simulink model "neuronchainv9"  (Simulink 7.6)
settings: Solver=ode45  StartTime=0.0  StopTime=250  RelTol=1e-3
values from neighbouring files (symbols this model uses):
  alpha = 0.8    [excneuroninit.m]
  amp = 1    [excneuroninit.m]
  ampe = 0.5    [excneuroninit.m]
  beta = 0.05    [excneuroninit.m]
  h = -0.5    [excneuroninit.m]
  inittime = 0.1    [excneuroninit.m]
  maxcon = 10    [excneuroninit.m]
  neurodist = 1    [excneuroninit.m]
  offsetf = 4    [excneuroninit.m]
  offseth = 4    [excneuroninit.m]
  offsetvali = 0    [excneuroninit.m]
  sensoryamp = 0.5    [excneuroninit.m]
  sigslope = 3    [excneuroninit.m]
  sigslopef = 1    [excneuroninit.m]
  sigslopei = 3    [excneuroninit.m]
  tau_s = 5    [excneuroninit.m]
  tau_u = 0.05    [excneuroninit.m]
  tau_v = 0.1    [excneuroninit.m]
  vari = 0.5    [excneuroninit.m]
undefined symbols (not defined in the file or in its folder): sigslopecal
libraries: simulink x560
(part 2 of 21)

== system neuronchainv9/signalsplit1k ==
  Mux : Mux  Inputs=5
  Pulse Generator{1..5} (5 alike) : DiscretePulseGenerator  PulseType=Time based  Period=4  PulseWidth=25  PhaseDelay=0.75
  Random Number{1..5} (5 alike) : RandomNumber  Variance=vari  SampleTime=0.1
  Sum{1..5} (5 alike) : Sum  Inputs=|++
  Out1 : Outport
  wires:
  Random Number1/1 -> Sum1/1
  Pulse Generator1/1 -> Sum1/2
  Random Number2/1 -> Sum2/1
  Pulse Generator2/1 -> Sum2/2
  Random Number3/1 -> Sum3/1
  Pulse Generator3/1 -> Sum3/2
  Random Number4/1 -> Sum4/1
  Pulse Generator4/1 -> Sum4/2
  Random Number5/1 -> Sum5/1
  Pulse Generator5/1 -> Sum5/2
  Sum5/1 -> Mux/1
  Sum2/1 -> Mux/2
  Sum1/1 -> Mux/3
  Sum4/1 -> Mux/4
  Sum3/1 -> Mux/5
  Mux/1 -> Out1/1

== system neuronchainv9/signalsplit2 ==
  Mux : Mux  Inputs=5
  Pulse Generator{1..5} (5 alike) : DiscretePulseGenerator  PulseType=Time based  Period=4  PulseWidth=25  PhaseDelay=1.35
  Random Number{1..5} (5 alike) : RandomNumber  Variance=vari  SampleTime=0.1
  Sum{1..5} (5 alike) : Sum  Inputs=|++
  Out1 : Outport
  wires:
  Pulse Generator2/1 -> Sum2/2
  Random Number2/1 -> Sum2/1
  Pulse Generator1/1 -> Sum1/2
  Random Number1/1 -> Sum1/1
  Pulse Generator3/1 -> Sum3/2
  Random Number3/1 -> Sum3/1
  Pulse Generator4/1 -> Sum4/2
  Random Number4/1 -> Sum4/1
  Pulse Generator5/1 -> Sum5/2
  Random Number5/1 -> Sum5/1
  Sum2/1 -> Mux/1
  Sum3/1 -> Mux/2
  Sum5/1 -> Mux/3
  Sum1/1 -> Mux/4
  Sum4/1 -> Mux/5
  Mux/1 -> Out1/1

== system neuronchainv9/signalsplit2k ==
  Mux : Mux  Inputs=5
  Pulse Generator{1..5} (5 alike) : DiscretePulseGenerator  PulseType=Time based  Period=4  PulseWidth=25  PhaseDelay=2.75
  Random Number{1..5} (5 alike) : RandomNumber  Variance=vari  SampleTime=0.1
  Sum{1..5} (5 alike) : Sum  Inputs=|++
  Out1 : Outport
  wires:
  Pulse Generator2/1 -> Sum2/2
  Random Number2/1 -> Sum2/1
  Pulse Generator1/1 -> Sum1/2
  Random Number1/1 -> Sum1/1
  Pulse Generator3/1 -> Sum3/2
  Random Number3/1 -> Sum3/1
  Pulse Generator4/1 -> Sum4/2
  Random Number4/1 -> Sum4/1
  Pulse Generator5/1 -> Sum5/2
  Random Number5/1 -> Sum5/1
  Sum2/1 -> Mux/1
  Sum3/1 -> Mux/2
  Sum5/1 -> Mux/3
  Sum1/1 -> Mux/4
  Sum4/1 -> Mux/5
  Mux/1 -> Out1/1

== system neuronchainv9/EAT_POOL/Subsystem ==
  port1 : Inport
  port2 : Inport  Port=2
  Add : Sum  Inputs=-+
  Demux : Demux  Outputs=2
  Gain : Gain  Gain=1/tau_v
  Integrator : Integrator  InitialCondition=1
  MATLAB Fcn : MATLABFcn  MATLABFcn=ampe*exp(-1*(u-neurodist).*(u-neurodist)/18)  OutputDimensions=maxcon
  Product1 : Product  RndMeth=Zero
  Product2 : Product  RndMeth=Zero
  Sigmoid1 : Subsystem  TreatAsAtomicUnit=on  mask{slope=sigslope, offset=offsetvali}
      mask help: Created by Han, Cheol
      mask Display: plot([10 15 20 25 30 35 40 45 50], [0 0.01 0.1 0.2 0.5 0.8 0.9 0.99 1])
  Sigmoid2 : Subsystem  TreatAsAtomicUnit=on  mask{slope=sigslope, offset=offsetvali}
      mask help: Created by Han, Cheol
      mask Display: plot([10 15 20 25 30 35 40 45 50], [0 0.01 0.1 0.2 0.5 0.8 0.9 0.99 1])
  Sum2 : Sum  Inputs=+  SaturateOnIntegerOverflow=on
  Out2 : Outport
  wires:
  Product2/1 -> Add/2
  port2/1 -> Sigmoid2/1(x)
  port1/1 -> MATLAB Fcn/1
  Sigmoid1/1(y) -> Product2/2
  Sum2/1 -> Product2/1
  Product1/1 -> Sum2/1
  Sigmoid2/1(y) -> Product1/2
  MATLAB Fcn/1 -> Product1/1
  Integrator/1 -> Out2/1, Sigmoid1/1(x), Add/1
  Gain/1 -> Integrator/1
  Add/1 -> Gain/1

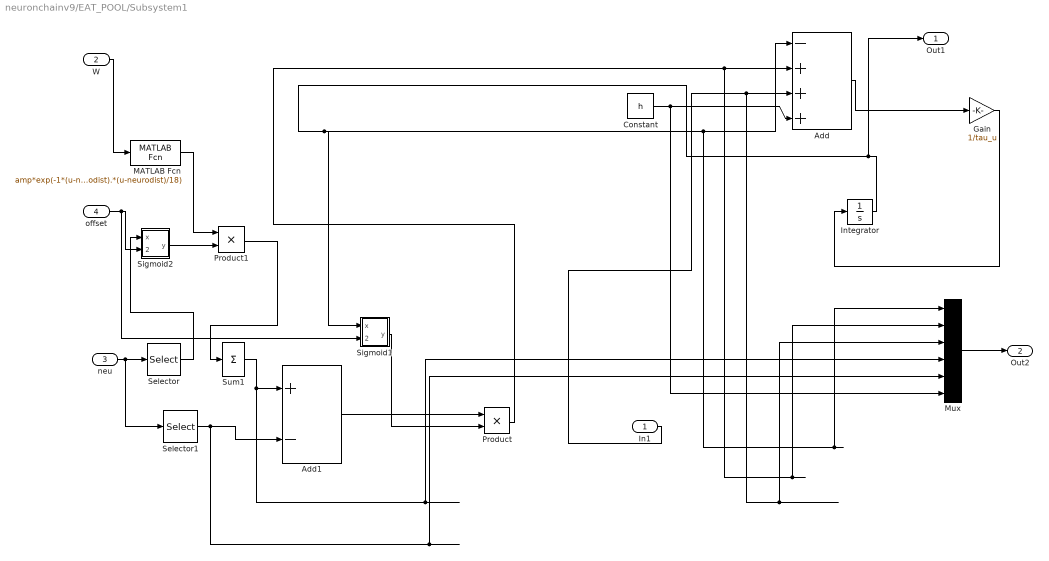
== system neuronchainv9/EAT_POOL/Subsystem1 ==
  In1 : Inport
  W : Inport  Port=2
  neu : Inport  Port=3
  offset : Inport  Port=4
  Add : Sum  Inputs=-+++
  Add1 : Sum  Inputs=+-
  Constant : Constant  Value=h
  Gain : Gain  Gain=1/tau_u
  Integrator : Integrator  InitialCondition=1
  MATLAB Fcn : MATLABFcn  MATLABFcn=amp*exp(-1*(u-neurodist).*(u-neurodist)/18)  OutputDimensions=maxcon
  Mux : Mux  Inputs=6
  Product : Product  RndMeth=Zero
  Product1 : Product  RndMeth=Zero
  Selector : Selector  InputPortWidth=maxcon+1  IndexOptions=Starting index (dialog)  Indices=2  OutputSizes=maxcon
  Selector1 : Selector  InputPortWidth=maxcon+1  IndexOptions=Starting index (dialog)  Indices=1  OutputSizes=1
  Sigmoid1 : Subsystem  TreatAsAtomicUnit=on  mask{slope=sigslope}
      mask help: Created by Han, Cheol
      mask Display: plot([10 15 20 25 30 35 40 45 50], [0 0.01 0.1 0.2 0.5 0.8 0.9 0.99 1])
  Sigmoid2 : Subsystem  TreatAsAtomicUnit=on  mask{slope=sigslope}
      mask help: Created by Han, Cheol
      mask Display: plot([10 15 20 25 30 35 40 45 50], [0 0.01 0.1 0.2 0.5 0.8 0.9 0.99 1])
  Sum1 : Sum  Inputs=+  SaturateOnIntegerOverflow=on
  Out1 : Outport
  Out2 : Outport  Port=2
  wires:
  Mux/1 -> Out2/1
  Constant/1 -> Mux/6, Add/4
  Add1/1 -> Product/2
  Sigmoid2/1(y) -> Product1/2
  Selector1/1 -> Mux/5, Add1/2
  Sum1/1 -> Mux/4, Add1/1
  MATLAB Fcn/1 -> Product1/1
  W/1 -> MATLAB Fcn/1
  Selector/1 -> Sigmoid2/1(x)
  Product1/1 -> Sum1/1
  neu/1 -> Selector1/1, Selector/1
  In1/1 -> Mux/3, Add/3
  Product/1 -> Mux/2, Add/2
  Sigmoid1/1(y) -> Product/1
  Integrator/1 -> Out1/1, Add/1, Mux/1, Sigmoid1/1(x)
  Gain/1 -> Integrator/1
  Add/1 -> Gain/1
  offset/1 -> Sigmoid2/2(offset), Sigmoid1/2(offset)

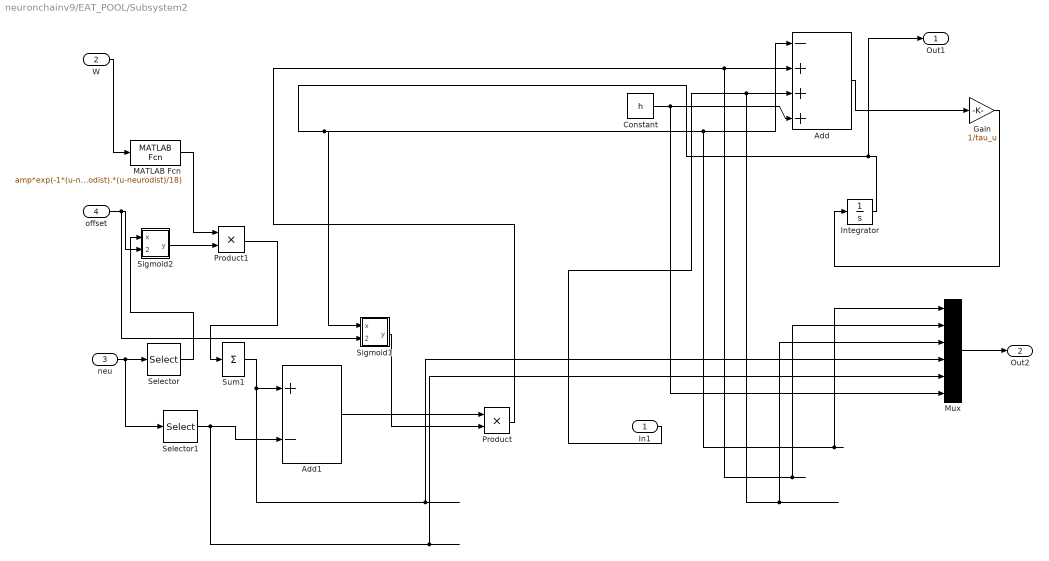
== system neuronchainv9/EAT_POOL/Subsystem2 ==
  In1 : Inport
  W : Inport  Port=2
  neu : Inport  Port=3
  offset : Inport  Port=4
  Add : Sum  Inputs=-+++
  Add1 : Sum  Inputs=+-
  Constant : Constant  Value=h
  Gain : Gain  Gain=1/tau_u
  Integrator : Integrator  InitialCondition=1
  MATLAB Fcn : MATLABFcn  MATLABFcn=amp*exp(-1*(u-neurodist).*(u-neurodist)/18)  OutputDimensions=maxcon
  Mux : Mux  Inputs=6
  Product : Product  RndMeth=Zero
  Product1 : Product  RndMeth=Zero
  Selector : Selector  InputPortWidth=maxcon+1  IndexOptions=Starting index (dialog)  Indices=2  OutputSizes=maxcon
  Selector1 : Selector  InputPortWidth=maxcon+1  IndexOptions=Starting index (dialog)  Indices=1  OutputSizes=1
  Sigmoid1 : Subsystem  TreatAsAtomicUnit=on  mask{slope=sigslope}
      mask help: Created by Han, Cheol
      mask Display: plot([10 15 20 25 30 35 40 45 50], [0 0.01 0.1 0.2 0.5 0.8 0.9 0.99 1])
  Sigmoid2 : Subsystem  TreatAsAtomicUnit=on  mask{slope=sigslope}
      mask help: Created by Han, Cheol
      mask Display: plot([10 15 20 25 30 35 40 45 50], [0 0.01 0.1 0.2 0.5 0.8 0.9 0.99 1])
  Sum1 : Sum  Inputs=+  SaturateOnIntegerOverflow=on
  Out1 : Outport
  Out2 : Outport  Port=2
  wires:
  Mux/1 -> Out2/1
  Constant/1 -> Mux/6, Add/4
  Add1/1 -> Product/2
  Sigmoid2/1(y) -> Product1/2
  Selector1/1 -> Mux/5, Add1/2
  Sum1/1 -> Mux/4, Add1/1
  MATLAB Fcn/1 -> Product1/1
  W/1 -> MATLAB Fcn/1
  Selector/1 -> Sigmoid2/1(x)
  Product1/1 -> Sum1/1
  neu/1 -> Selector1/1, Selector/1
  In1/1 -> Mux/3, Add/3
  Product/1 -> Mux/2, Add/2
  Sigmoid1/1(y) -> Product/1
  Integrator/1 -> Out1/1, Add/1, Mux/1, Sigmoid1/1(x)
  Gain/1 -> Integrator/1
  Add/1 -> Gain/1
  offset/1 -> Sigmoid2/2(offset), Sigmoid1/2(offset)

== system neuronchainv9/EAT_POOL/Subsystem3 ==
  In1 : Inport
  W : Inport  Port=2
  neu : Inport  Port=3
  offset : Inport  Port=4
  Add : Sum  Inputs=-+++
  Add1 : Sum  Inputs=+-
  Constant : Constant  Value=h
  Gain : Gain  Gain=1/tau_u
  Integrator : Integrator  InitialCondition=1
  MATLAB Fcn : MATLABFcn  MATLABFcn=amp*exp(-1*(u-neurodist).*(u-neurodist)/18)  OutputDimensions=maxcon
  Mux : Mux  Inputs=6
  Product : Product  RndMeth=Zero
  Product1 : Product  RndMeth=Zero
  Selector : Selector  InputPortWidth=maxcon+1  IndexOptions=Starting index (dialog)  Indices=2  OutputSizes=maxcon
  Selector1 : Selector  InputPortWidth=maxcon+1  IndexOptions=Starting index (dialog)  Indices=1  OutputSizes=1
  Sigmoid1 : Subsystem  TreatAsAtomicUnit=on  mask{slope=sigslope}
      mask help: Created by Han, Cheol
      mask Display: plot([10 15 20 25 30 35 40 45 50], [0 0.01 0.1 0.2 0.5 0.8 0.9 0.99 1])
  Sigmoid2 : Subsystem  TreatAsAtomicUnit=on  mask{slope=sigslope}
      mask help: Created by Han, Cheol
      mask Display: plot([10 15 20 25 30 35 40 45 50], [0 0.01 0.1 0.2 0.5 0.8 0.9 0.99 1])
  Sum1 : Sum  Inputs=+  SaturateOnIntegerOverflow=on
  Out1 : Outport
  Out2 : Outport  Port=2
  wires:
  Mux/1 -> Out2/1
  Constant/1 -> Mux/6, Add/4
  Add1/1 -> Product/2
  Sigmoid2/1(y) -> Product1/2
  Selector1/1 -> Mux/5, Add1/2
  Sum1/1 -> Mux/4, Add1/1
  MATLAB Fcn/1 -> Product1/1
  W/1 -> MATLAB Fcn/1
  Selector/1 -> Sigmoid2/1(x)
  Product1/1 -> Sum1/1
  neu/1 -> Selector1/1, Selector/1
  In1/1 -> Mux/3, Add/3
  Product/1 -> Mux/2, Add/2
  Sigmoid1/1(y) -> Product/1
  Integrator/1 -> Out1/1, Add/1, Mux/1, Sigmoid1/1(x)
  Gain/1 -> Integrator/1
  Add/1 -> Gain/1
  offset/1 -> Sigmoid2/2(offset), Sigmoid1/2(offset)

== system neuronchainv9/EAT_POOL/Subsystem4 ==
  port1 : Inport
  port2 : Inport  Port=2
  Add : Sum  Inputs=-+
  Gain : Gain  Gain=1/tau_v
  Integrator : Integrator  InitialCondition=1
  MATLAB Fcn : MATLABFcn  MATLABFcn=ampe*exp(-1*(u-neurodist).*(u-neurodist)/18)  OutputDimensions=maxcon
  Product1 : Product  RndMeth=Zero
  Product2 : Product  RndMeth=Zero
  Sigmoid1 : Subsystem  TreatAsAtomicUnit=on  mask{slope=sigslope, offset=offsetvali}
      mask help: Created by Han, Cheol
      mask Display: plot([10 15 20 25 30 35 40 45 50], [0 0.01 0.1 0.2 0.5 0.8 0.9 0.99 1])
  Sigmoid2 : Subsystem  TreatAsAtomicUnit=on  mask{slope=sigslope, offset=offsetvali}
      mask help: Created by Han, Cheol
      mask Display: plot([10 15 20 25 30 35 40 45 50], [0 0.01 0.1 0.2 0.5 0.8 0.9 0.99 1])
  Sum2 : Sum  Inputs=+  SaturateOnIntegerOverflow=on
  Out2 : Outport
  wires:
  Product2/1 -> Add/2
  port2/1 -> Sigmoid2/1(x)
  port1/1 -> MATLAB Fcn/1
  Sigmoid1/1(y) -> Product2/2
  Sum2/1 -> Product2/1
  Product1/1 -> Sum2/1
  Sigmoid2/1(y) -> Product1/2
  MATLAB Fcn/1 -> Product1/1
  Integrator/1 -> Out2/1, Sigmoid1/1(x), Add/1
  Gain/1 -> Integrator/1
  Add/1 -> Gain/1

== system neuronchainv9/EAT_POOL/Subsystem5 ==
  port1 : Inport
  port2 : Inport  Port=2
  Add : Sum  Inputs=-+
  Gain : Gain  Gain=1/tau_v
  Integrator : Integrator  InitialCondition=1
  MATLAB Fcn : MATLABFcn  MATLABFcn=ampe*exp(-1*(u-neurodist).*(u-neurodist)/18)  OutputDimensions=maxcon
  Product1 : Product  RndMeth=Zero
  Product2 : Product  RndMeth=Zero
  Sigmoid1 : Subsystem  TreatAsAtomicUnit=on  mask{slope=sigslope, offset=offsetvali}
      mask help: Created by Han, Cheol
      mask Display: plot([10 15 20 25 30 35 40 45 50], [0 0.01 0.1 0.2 0.5 0.8 0.9 0.99 1])
  Sigmoid2 : Subsystem  TreatAsAtomicUnit=on  mask{slope=sigslope, offset=offsetvali}
      mask help: Created by Han, Cheol
      mask Display: plot([10 15 20 25 30 35 40 45 50], [0 0.01 0.1 0.2 0.5 0.8 0.9 0.99 1])
  Sum2 : Sum  Inputs=+  SaturateOnIntegerOverflow=on
  Out2 : Outport
  wires:
  Product2/1 -> Add/2
  port2/1 -> Sigmoid2/1(x)
  port1/1 -> MATLAB Fcn/1
  Sigmoid1/1(y) -> Product2/2
  Sum2/1 -> Product2/1
  Product1/1 -> Sum2/1
  Sigmoid2/1(y) -> Product1/2
  MATLAB Fcn/1 -> Product1/1
  Integrator/1 -> Out2/1, Sigmoid1/1(x), Add/1
  Gain/1 -> Integrator/1
  Add/1 -> Gain/1

== system neuronchainv9/EAT_POOL/Subsystem6 ==
  In1 : Inport
  W : Inport  Port=2
  neu : Inport  Port=3
  offset : Inport  Port=4
  Add : Sum  Inputs=-+++
  Add1 : Sum  Inputs=+-
  Constant : Constant  Value=h
  Gain : Gain  Gain=1/tau_u
  Integrator : Integrator  InitialCondition=1
  MATLAB Fcn : MATLABFcn  MATLABFcn=amp*exp(-1*(u-neurodist).*(u-neurodist)/18)  OutputDimensions=maxcon
  Mux : Mux  Inputs=6
  Product : Product  RndMeth=Zero
  Product1 : Product  RndMeth=Zero
  Selector : Selector  InputPortWidth=maxcon+1  IndexOptions=Starting index (dialog)  Indices=2  OutputSizes=maxcon
  Selector1 : Selector  InputPortWidth=maxcon+1  IndexOptions=Starting index (dialog)  Indices=1  OutputSizes=1
  Sigmoid1 : Subsystem  TreatAsAtomicUnit=on  mask{slope=sigslope}
      mask help: Created by Han, Cheol
      mask Display: plot([10 15 20 25 30 35 40 45 50], [0 0.01 0.1 0.2 0.5 0.8 0.9 0.99 1])
  Sigmoid2 : Subsystem  TreatAsAtomicUnit=on  mask{slope=sigslope}
      mask help: Created by Han, Cheol
      mask Display: plot([10 15 20 25 30 35 40 45 50], [0 0.01 0.1 0.2 0.5 0.8 0.9 0.99 1])
  Sum1 : Sum  Inputs=+  SaturateOnIntegerOverflow=on
  Out1 : Outport
  Out2 : Outport  Port=2
  wires:
  Mux/1 -> Out2/1
  Constant/1 -> Mux/6, Add/4
  Add1/1 -> Product/2
  Sigmoid2/1(y) -> Product1/2
  Selector1/1 -> Mux/5, Add1/2
  Sum1/1 -> Mux/4, Add1/1
  MATLAB Fcn/1 -> Product1/1
  W/1 -> MATLAB Fcn/1
  Selector/1 -> Sigmoid2/1(x)
  Product1/1 -> Sum1/1
  neu/1 -> Selector1/1, Selector/1
  In1/1 -> Mux/3, Add/3
  Product/1 -> Mux/2, Add/2
  Sigmoid1/1(y) -> Product/1
  Integrator/1 -> Out1/1, Add/1, Mux/1, Sigmoid1/1(x)
  Gain/1 -> Integrator/1
  Add/1 -> Gain/1
  offset/1 -> Sigmoid2/2(offset), Sigmoid1/2(offset)

== system neuronchainv9/EAT_POOL/Subsystem7 ==
  In1 : Inport
  W : Inport  Port=2
  neu : Inport  Port=3
  offset : Inport  Port=4
  Add : Sum  Inputs=-+++
  Add1 : Sum  Inputs=+-
  Constant : Constant  Value=h
  Gain : Gain  Gain=1/tau_u
  Integrator : Integrator  InitialCondition=1
  MATLAB Fcn : MATLABFcn  MATLABFcn=amp*exp(-1*(u-neurodist).*(u-neurodist)/18)  OutputDimensions=maxcon
  Mux : Mux  Inputs=6
  Product : Product  RndMeth=Zero
  Product1 : Product  RndMeth=Zero
  Selector : Selector  InputPortWidth=maxcon+1  IndexOptions=Starting index (dialog)  Indices=2  OutputSizes=maxcon
  Selector1 : Selector  InputPortWidth=maxcon+1  IndexOptions=Starting index (dialog)  Indices=1  OutputSizes=1
  Sigmoid1 : Subsystem  TreatAsAtomicUnit=on  mask{slope=sigslope}
      mask help: Created by Han, Cheol
      mask Display: plot([10 15 20 25 30 35 40 45 50], [0 0.01 0.1 0.2 0.5 0.8 0.9 0.99 1])
  Sigmoid2 : Subsystem  TreatAsAtomicUnit=on  mask{slope=sigslope}
      mask help: Created by Han, Cheol
      mask Display: plot([10 15 20 25 30 35 40 45 50], [0 0.01 0.1 0.2 0.5 0.8 0.9 0.99 1])
  Sum1 : Sum  Inputs=+  SaturateOnIntegerOverflow=on
  Out1 : Outport
  Out2 : Outport  Port=2
  wires:
  Mux/1 -> Out2/1
  Constant/1 -> Mux/6, Add/4
  Add1/1 -> Product/2
  Sigmoid2/1(y) -> Product1/2
  Selector1/1 -> Mux/5, Add1/2
  Sum1/1 -> Mux/4, Add1/1
  MATLAB Fcn/1 -> Product1/1
  W/1 -> MATLAB Fcn/1
  Selector/1 -> Sigmoid2/1(x)
  Product1/1 -> Sum1/1
  neu/1 -> Selector1/1, Selector/1
  In1/1 -> Mux/3, Add/3
  Product/1 -> Mux/2, Add/2
  Sigmoid1/1(y) -> Product/1
  Integrator/1 -> Out1/1, Add/1, Mux/1, Sigmoid1/1(x)
  Gain/1 -> Integrator/1
  Add/1 -> Gain/1
  offset/1 -> Sigmoid2/2(offset), Sigmoid1/2(offset)

== system neuronchainv9/EAT_POOL/Subsystem8 ==
  port1 : Inport
  port2 : Inport  Port=2
  Add : Sum  Inputs=-+
  Gain : Gain  Gain=1/tau_v
  Integrator : Integrator  InitialCondition=1
  MATLAB Fcn : MATLABFcn  MATLABFcn=ampe*exp(-1*(u-neurodist).*(u-neurodist)/18)  OutputDimensions=maxcon
  Product1 : Product  RndMeth=Zero
  Product2 : Product  RndMeth=Zero
  Sigmoid1 : Subsystem  TreatAsAtomicUnit=on  mask{slope=sigslope, offset=offsetvali}
      mask help: Created by Han, Cheol
      mask Display: plot([10 15 20 25 30 35 40 45 50], [0 0.01 0.1 0.2 0.5 0.8 0.9 0.99 1])
  Sigmoid2 : Subsystem  TreatAsAtomicUnit=on  mask{slope=sigslope, offset=offsetvali}
      mask help: Created by Han, Cheol
      mask Display: plot([10 15 20 25 30 35 40 45 50], [0 0.01 0.1 0.2 0.5 0.8 0.9 0.99 1])
  Sum2 : Sum  Inputs=+  SaturateOnIntegerOverflow=on
  Out2 : Outport
  wires:
  Product2/1 -> Add/2
  port2/1 -> Sigmoid2/1(x)
  port1/1 -> MATLAB Fcn/1
  Sigmoid1/1(y) -> Product2/2
  Sum2/1 -> Product2/1
  Product1/1 -> Sum2/1
  Sigmoid2/1(y) -> Product1/2
  MATLAB Fcn/1 -> Product1/1
  Integrator/1 -> Out2/1, Sigmoid1/1(x), Add/1
  Gain/1 -> Integrator/1
  Add/1 -> Gain/1

== system neuronchainv9/EAT_POOL/Subsystem9 ==
  port1 : Inport
  port2 : Inport  Port=2
  Add : Sum  Inputs=-+
  Gain : Gain  Gain=1/tau_v
  Integrator : Integrator  InitialCondition=1
  MATLAB Fcn : MATLABFcn  MATLABFcn=ampe*exp(-1*(u-neurodist).*(u-neurodist)/18)  OutputDimensions=maxcon
  Product1 : Product  RndMeth=Zero
  Product2 : Product  RndMeth=Zero
  Sigmoid1 : Subsystem  TreatAsAtomicUnit=on  mask{slope=sigslope, offset=offsetvali}
      mask help: Created by Han, Cheol
      mask Display: plot([10 15 20 25 30 35 40 45 50], [0 0.01 0.1 0.2 0.5 0.8 0.9 0.99 1])
  Sigmoid2 : Subsystem  TreatAsAtomicUnit=on  mask{slope=sigslope, offset=offsetvali}
      mask help: Created by Han, Cheol
      mask Display: plot([10 15 20 25 30 35 40 45 50], [0 0.01 0.1 0.2 0.5 0.8 0.9 0.99 1])
  Sum2 : Sum  Inputs=+  SaturateOnIntegerOverflow=on
  Out2 : Outport
  wires:
  Product2/1 -> Add/2
  port2/1 -> Sigmoid2/1(x)
  port1/1 -> MATLAB Fcn/1
  Sigmoid1/1(y) -> Product2/2
  Sum2/1 -> Product2/1
  Product1/1 -> Sum2/1
  Sigmoid2/1(y) -> Product1/2
  MATLAB Fcn/1 -> Product1/1
  Integrator/1 -> Out2/1, Sigmoid1/1(x), Add/1
  Gain/1 -> Integrator/1
  Add/1 -> Gain/1

== system neuronchainv9/Hebbconp0to1/  ==
  ui : Inport
  uj : Inport  Port=2
  Sij : Inport  Port=3
  1_over_taus : Gain  Gain=1/tau_s
  Clock : Clock
      settings: Decimation=10
  Constant : Constant  Value=alpha
  Constant3 : Constant  Value=inittime
  Integrator : Integrator
  Product : Product  Inputs=3  RndMeth=Zero
  Product1 : Product  RndMeth=Zero
  Sum : Sum  Inputs=|+-
  Sum1 : Sum  Inputs=|+-
  Sum2 : Sum  Inputs=+-|
  Switch1 : Switch  Criteria=u2 > Threshold
  beta : Gain  Gain=beta
  f(ui) : Subsystem  TreatAsAtomicUnit=on  mask{slope=sigslopef, offset=offsetf}
      mask help: Created by Han, Cheol
      mask Display: plot([10 15 20 25 30 35 40 45 50], [0 0.01 0.1 0.2 0.5 0.8 0.9 0.99 1])
  f(uj) : Subsystem  TreatAsAtomicUnit=on  mask{slope=sigslopef, offset=offsetf}
      mask help: Created by Han, Cheol
      mask Display: plot([10 15 20 25 30 35 40 45 50], [0 0.01 0.1 0.2 0.5 0.8 0.9 0.99 1])
  h(ui) : Subsystem  TreatAsAtomicUnit=on  mask{slope=sigslopei, offset=offseth}
      mask help: Created by Han, Cheol
      mask Display: plot([10 15 20 25 30 35 40 45 50], [0 0.01 0.1 0.2 0.5 0.8 0.9 0.99 1])
  Out1 : Outport
  wires:
  ui/1 -> h(ui)/1(x), f(ui)/1(x)
  uj/1 -> f(uj)/1(x)
  f(ui)/1(y) -> Product/1
  f(uj)/1(y) -> Product/2
  Constant/1 -> Product/3
  Product/1 -> Sum/1
  Sum/1 -> Product1/2
  h(ui)/1(y) -> Product1/1
  Product1/1 -> Sum1/1
  beta/1 -> Sum1/2
  Sum1/1 -> 1_over_taus/1
  1_over_taus/1 -> Integrator/1
  Integrator/1 -> Switch1/1, Sum/2
  Sum2/1 -> Switch1/2
  Clock/1 -> Sum2/1
  Constant3/1 -> Sum2/2
  Switch1/1 -> beta/1, Out1/1
  Sij/1 -> Switch1/3

== system neuronchainv9/Hebbconp0to1/ 2 ==
  ui : Inport
  uj : Inport  Port=2
  Sij : Inport  Port=3
  1_over_taus : Gain  Gain=1/tau_s
  Clock : Clock
      settings: Decimation=10
  Constant : Constant  Value=alpha
  Constant3 : Constant  Value=inittime
  Integrator : Integrator
  Product : Product  Inputs=3  RndMeth=Zero
  Product1 : Product  RndMeth=Zero
  Sum : Sum  Inputs=|+-
  Sum1 : Sum  Inputs=|+-
  Sum2 : Sum  Inputs=+-|
  Switch1 : Switch  Criteria=u2 > Threshold
  beta : Gain  Gain=beta
  f(ui) : Subsystem  TreatAsAtomicUnit=on  mask{slope=sigslopef, offset=offsetf}
      mask help: Created by Han, Cheol
      mask Display: plot([10 15 20 25 30 35 40 45 50], [0 0.01 0.1 0.2 0.5 0.8 0.9 0.99 1])
  f(uj) : Subsystem  TreatAsAtomicUnit=on  mask{slope=sigslopef, offset=offsetf}
      mask help: Created by Han, Cheol
      mask Display: plot([10 15 20 25 30 35 40 45 50], [0 0.01 0.1 0.2 0.5 0.8 0.9 0.99 1])
  h(ui) : Subsystem  TreatAsAtomicUnit=on  mask{slope=sigslopei, offset=offseth}
      mask help: Created by Han, Cheol
      mask Display: plot([10 15 20 25 30 35 40 45 50], [0 0.01 0.1 0.2 0.5 0.8 0.9 0.99 1])
  Out1 : Outport
  wires:
  ui/1 -> h(ui)/1(x), f(ui)/1(x)
  uj/1 -> f(uj)/1(x)
  f(ui)/1(y) -> Product/1
  f(uj)/1(y) -> Product/2
  Constant/1 -> Product/3
  Product/1 -> Sum/1
  Sum/1 -> Product1/2
  h(ui)/1(y) -> Product1/1
  Product1/1 -> Sum1/1
  beta/1 -> Sum1/2
  Sum1/1 -> 1_over_taus/1
  1_over_taus/1 -> Integrator/1
  Integrator/1 -> Switch1/1, Sum/2
  Sum2/1 -> Switch1/2
  Clock/1 -> Sum2/1
  Constant3/1 -> Sum2/2
  Switch1/1 -> beta/1, Out1/1
  Sij/1 -> Switch1/3

== system neuronchainv9/Hebbconp0to1/11 ==
  ui : Inport
  uj : Inport  Port=2
  Sij : Inport  Port=3
  1_over_taus : Gain  Gain=1/tau_s
  Clock : Clock
      settings: Decimation=10
  Constant : Constant  Value=alpha
  Constant3 : Constant  Value=inittime
  Integrator : Integrator
  Product : Product  Inputs=3  RndMeth=Zero
  Product1 : Product  RndMeth=Zero
  Sum : Sum  Inputs=|+-
  Sum1 : Sum  Inputs=|+-
  Sum2 : Sum  Inputs=+-|
  Switch1 : Switch  Criteria=u2 > Threshold
  beta : Gain  Gain=beta
  f(ui) : Subsystem  TreatAsAtomicUnit=on  mask{slope=sigslopef, offset=offsetf}
      mask help: Created by Han, Cheol
      mask Display: plot([10 15 20 25 30 35 40 45 50], [0 0.01 0.1 0.2 0.5 0.8 0.9 0.99 1])
  f(uj) : Subsystem  TreatAsAtomicUnit=on  mask{slope=sigslopef, offset=offsetf}
      mask help: Created by Han, Cheol
      mask Display: plot([10 15 20 25 30 35 40 45 50], [0 0.01 0.1 0.2 0.5 0.8 0.9 0.99 1])
  h(ui) : Subsystem  TreatAsAtomicUnit=on  mask{slope=sigslopei, offset=offseth}
      mask help: Created by Han, Cheol
      mask Display: plot([10 15 20 25 30 35 40 45 50], [0 0.01 0.1 0.2 0.5 0.8 0.9 0.99 1])
  Out1 : Outport
  wires:
  ui/1 -> h(ui)/1(x), f(ui)/1(x)
  uj/1 -> f(uj)/1(x)
  f(ui)/1(y) -> Product/1
  f(uj)/1(y) -> Product/2
  Constant/1 -> Product/3
  Product/1 -> Sum/1
  Sum/1 -> Product1/2
  h(ui)/1(y) -> Product1/1
  Product1/1 -> Sum1/1
  beta/1 -> Sum1/2
  Sum1/1 -> 1_over_taus/1
  1_over_taus/1 -> Integrator/1
  Integrator/1 -> Switch1/1, Sum/2
  Sum2/1 -> Switch1/2
  Clock/1 -> Sum2/1
  Constant3/1 -> Sum2/2
  Switch1/1 -> beta/1, Out1/1
  Sij/1 -> Switch1/3
